simulink model "simulation_environment_thesis"  (R2024b)
settings: Solver=VariableStepAuto  StartTime=0.0  StopTime=3200  RelTol=1e-3
values from neighbouring files (symbols this model uses):
  A = [1, dt; 0, 1]    [estimate_and_control_fully_actuated_with_current.m]
  A1 = [1, 0, 0, 0, dt, 0 ... (first row only)    [estimate_and_control_fully_actuated_with_current.m]
  alpha = 10    [estimate_and_control_fully_actuated_with_current.m]
  alpha_xy_1 = 1    [estimate_and_control_fully_actuated_with_current.m]
  alpha_z_1 = 5    [estimate_and_control_fully_actuated_with_current.m]
  Az1 = [1, dt ... (first row only)    [estimate_and_control_fully_actuated_with_current.m]
  B = [dt; 0]    [estimate_and_control_fully_actuated_with_current.m]
  B1 = [dt, 0 ... (first row only)    [estimate_and_control_fully_actuated_with_current.m]
  Bz1 = [dt ... (first row only)    [estimate_and_control_fully_actuated_with_current.m]
  C1 = [1, 0, 0, 0, 0, 0 ... (first row only)    [estimate_and_control_fully_actuated_with_current.m]
  circumference_x = min_dist_from_ds*cos(theta)    [estimate_and_control_fully_actuated_with_current.m]
  circumference_y = min_dist_from_ds*sin(theta)    [estimate_and_control_fully_actuated_with_current.m]
  const_current = [-r; -s; 0; 0]    [estimate_and_control_fully_actuated_with_current.m]
  csi_psi_1 = 0.0003    [estimate_and_control_fully_actuated_with_current.m]
  csi_r_1 = 0.0003    [estimate_and_control_fully_actuated_with_current.m]
  csi_vcx_1 = 0.0003    [estimate_and_control_fully_actuated_with_current.m]
  csi_vcy_1 = 0.0003    [estimate_and_control_fully_actuated_with_current.m]
  csi_vcz_1 = 0.005    [estimate_and_control_fully_actuated_with_current.m]
  csi_x_1 = 0.003    [estimate_and_control_fully_actuated_with_current.m]
  csi_y_1 = 0.003    [estimate_and_control_fully_actuated_with_current.m]
  csi_z_1 = 0.005    [estimate_and_control_fully_actuated_with_current.m]
  Cz1 = [1, 0]    [estimate_and_control_fully_actuated_with_current.m]
  d = 1    [estimate_and_control_fully_actuated_with_current.m]
  delta_FOV = pi/7    (= 0.448798950513)    [estimate_and_control_fully_actuated_with_current.m]
  desired_speed = speed_0    [estimate_and_control_fully_actuated_with_current.m]
  dt = 1/Tsim    (= 0.005)    [estimate_and_control_fully_actuated_with_current.m]
  eta1 = deg2rad(0.5)    [estimate_and_control_fully_actuated_with_current.m]
  eta2 = deg2rad(0.5)    [estimate_and_control_fully_actuated_with_current.m]
  eta_psi_1 = 0.003    [estimate_and_control_fully_actuated_with_current.m]
  eta_x_1 = 0.03    [estimate_and_control_fully_actuated_with_current.m]
  eta_y_1 = 0.03    [estimate_and_control_fully_actuated_with_current.m]
  eta_z_1 = 0.005    [estimate_and_control_fully_actuated_with_current.m]
  G = dt*eye(2)    [estimate_and_control_fully_actuated_with_current.m]
  G1 = dt*eye(6)    [estimate_and_control_fully_actuated_with_current.m]
  Gz1 = dt*eye(2)    [estimate_and_control_fully_actuated_with_current.m]
  left_reference = L_x*[cos(NED_psi_ds); sin(NED_psi_ds); 0] + L_y_l*[-sin(NED_psi_ds); cos(NED_psi_ds); 0]    [estimate_and_control_fully_actuated_with_current.m]
  max_dist_from_ship = 60    [estimate_and_control_fully_actuated_with_current.m]
  min_dist_from_ds = 45    [estimate_and_control_fully_actuated_with_current.m]
  mu = -5    [estimate_and_control_fully_actuated_with_current.m]
  NED_psi_ds = -pi/2    (= -1.57079632679)    [estimate_and_control_fully_actuated_with_current.m]
  p_b1 = [-90; 90; 5]    [estimate_and_control_fully_actuated_with_current.m]
  position_t_0 = [0; 0; 0; 0]    [estimate_and_control_fully_actuated_with_current.m]
  psi_P = linspace(0,2*pi,360)'    [estimate_and_control_fully_actuated_with_current.m]
  Q = [csi1_kf^2, 0 ... (first row only)    [estimate_and_control_fully_actuated_with_current.m]
  Q1 = [csi_x_1_kf^2, 0, 0, 0, 0, 0 ... (first row only)    [estimate_and_control_fully_actuated_with_current.m]
  Qz1 = [csi_z_1_kf^2, 0 ... (first row only)    [estimate_and_control_fully_actuated_with_current.m]
  R = [eta1_kf^2, 0 ... (first row only)    [estimate_and_control_fully_actuated_with_current.m]
  R1 = [eta_x_1_kf^2, 0, 0 ... (first row only)    [estimate_and_control_fully_actuated_with_current.m]
  right_reference = L_x*[cos(NED_psi_ds); sin(NED_psi_ds); 0] + L_y_r*[sin(NED_psi_ds); -cos(NED_psi_ds); 0]    [estimate_and_control_fully_actuated_with_current.m]
  Rz1 = eta_z_1_kf^2    (= 1e-06)    [estimate_and_control_fully_actuated_with_current.m]
  speed_0 = [0.1, 0.1, 0, 0]'    [estimate_and_control_fully_actuated_with_current.m]
  tau_heave_sat = 30    [estimate_and_control_fully_actuated_with_current.m]
  tau_surge_sat = 10    [estimate_and_control_fully_actuated_with_current.m]
  tau_sway_sat = 30    [estimate_and_control_fully_actuated_with_current.m]
  tau_yaw_sat = 0.1    [estimate_and_control_fully_actuated_with_current.m]
  var_vm_x = 0.001    [estimate_and_control_fully_actuated_with_current.m]
  var_vm_y = 0.001    [estimate_and_control_fully_actuated_with_current.m]
  var_vm_z = 0.001    [estimate_and_control_fully_actuated_with_current.m]
undefined symbols (not defined in the file or in its folder): A2, alpha2, B2, csi1_2, csi2_2, csi3_2, csi4_2, csi5_2, csi6_2, csi7_2, csi8_2, eta1_2, eta2_2, eta3_2, eta4_2, eta5_2, eta6_2, G2, Q2, R2
libraries: simulink x60, simulink_extras x5
(part 7 of 16)

== system simulation_environment_thesis/PHASE 1.21/CONTROLLER1/ANTIWINDUP SURGE ==
  in : Inport
  K : Inport  Port=2
  K1 : Inport  Port=3
  K_t_u : Inport  Port=4
  Integrator21 : Integrator
  MATLAB Function21 : MATLAB Function  TreatAsAtomicUnit=on
  MATLAB Function22 : MATLAB Function  TreatAsAtomicUnit=on
  MATLAB Function23 : MATLAB Function  TreatAsAtomicUnit=on
  Saturation2 : Saturate  UpperLimit=tau_surge_sat  LowerLimit=-tau_surge_sat
  Sum11 : Sum  Inputs=|++
  Sum12 : Sum  Inputs=|+-
  Sum13 : Sum  Inputs=|++
  tau_u : Outport
  wires:
  in/1 -> MATLAB Function22/1(in), MATLAB Function21/1(in)
  Sum12/1 -> MATLAB Function23/1(e_s_u)
  Sum11/1 -> Integrator21/1
  Sum13/1 -> Saturation2/1, Sum12/2
  MATLAB Function22/1(y) -> Sum11/1
  MATLAB Function21/1(y) -> Sum13/1
  Saturation2/1 -> tau_u/1, Sum12/1
  K1/1 -> MATLAB Function21/2(K)
  K_t_u/1 -> MATLAB Function23/2(K_t_u)
  K/1 -> MATLAB Function22/2(K)
  MATLAB Function23/1(y) -> Sum11/2
  Integrator21/1 -> Sum13/2

== system simulation_environment_thesis/PHASE 1.21/CONTROLLER1/ANTIWINDUP SWAY ==
  in : Inport
  K : Inport  Port=2
  K1 : Inport  Port=3
  K_t_v : Inport  Port=4
  Integrator21 : Integrator
  MATLAB Function21 : MATLAB Function  TreatAsAtomicUnit=on
  MATLAB Function22 : MATLAB Function  TreatAsAtomicUnit=on
  MATLAB Function23 : MATLAB Function  TreatAsAtomicUnit=on
  Saturation2 : Saturate  UpperLimit=tau_sway_sat  LowerLimit=-tau_sway_sat
  Sum11 : Sum  Inputs=|++
  Sum12 : Sum  Inputs=|+-
  Sum13 : Sum  Inputs=|++
  tau_v : Outport
  wires:
  in/1 -> MATLAB Function22/1(in), MATLAB Function21/1(in)
  Sum12/1 -> MATLAB Function23/1(e_s_v)
  Sum11/1 -> Integrator21/1
  Sum13/1 -> Saturation2/1, Sum12/2
  MATLAB Function22/1(y) -> Sum11/1
  MATLAB Function21/1(y) -> Sum13/1
  Saturation2/1 -> tau_v/1, Sum12/1
  K1/1 -> MATLAB Function21/2(K)
  K_t_v/1 -> MATLAB Function23/2(K_t_v)
  K/1 -> MATLAB Function22/2(K)
  MATLAB Function23/1(y) -> Sum11/2
  Integrator21/1 -> Sum13/2

== system simulation_environment_thesis/PHASE 1.21/CONTROLLER1/ANTIWINDUP_HEAVE ==
  K1 : Inport
  K2 : Inport  Port=2
  K_t_w : Inport  Port=3
  in1 : Inport  Port=4
  in : Inport  Port=5
  K : Inport  Port=6
  Integrator2 : Integrator
  MATLAB Function11 : MATLAB Function  TreatAsAtomicUnit=on
  MATLAB Function14 : MATLAB Function  TreatAsAtomicUnit=on
  MATLAB Function15 : MATLAB Function  TreatAsAtomicUnit=on
  MATLAB Function16 : MATLAB Function  TreatAsAtomicUnit=on
  Saturation1 : Saturate  UpperLimit=tau_heave_sat  LowerLimit=-tau_heave_sat
  Sum4 : Sum  Inputs=|+-
  Sum8 : Sum  Inputs=|+++
  Sum9 : Sum  Inputs=|++
  tau_w : Outport
  wires:
  in/1 -> MATLAB Function16/1(in)
  MATLAB Function11/1(y) -> Sum9/2
  MATLAB Function14/1(y) -> Sum8/1
  MATLAB Function16/1(y) -> Sum8/3
  K_t_w/1 -> MATLAB Function11/2(K_t_w)
  MATLAB Function15/1(y) -> Sum9/1
  Integrator2/1 -> Sum8/2
  K/1 -> MATLAB Function16/2(K)
  K2/1 -> MATLAB Function14/2(K)
  K1/1 -> MATLAB Function15/2(K)
  Saturation1/1 -> tau_w/1, Sum4/1
  Sum4/1 -> MATLAB Function11/1(e_s_w)
  in1/1 -> MATLAB Function15/1(in), MATLAB Function14/1(in)
  Sum9/1 -> Integrator2/1
  Sum8/1 -> Sum4/2, Saturation1/1

== system simulation_environment_thesis/PHASE 1.21/CONTROLLER1/ANTIWINDUP_YAW ==
  K1 : Inport
  K2 : Inport  Port=2
  K_t_psi : Inport  Port=3
  in1 : Inport  Port=4
  in : Inport  Port=5
  K : Inport  Port=6
  Integrator2 : Integrator
  MATLAB Function11 : MATLAB Function  TreatAsAtomicUnit=on
  MATLAB Function14 : MATLAB Function  TreatAsAtomicUnit=on
  MATLAB Function15 : MATLAB Function  TreatAsAtomicUnit=on
  MATLAB Function16 : MATLAB Function  TreatAsAtomicUnit=on
  Saturation1 : Saturate  UpperLimit=tau_yaw_sat  LowerLimit=-tau_yaw_sat
  Sum4 : Sum  Inputs=|+-
  Sum8 : Sum  Inputs=|+++
  Sum9 : Sum  Inputs=|++
  tau_psi : Outport
  wires:
  MATLAB Function15/1(y) -> Sum9/1
  Sum8/1 -> Sum4/2, Saturation1/1
  in1/1 -> MATLAB Function15/1(in), MATLAB Function14/1(in)
  Saturation1/1 -> tau_psi/1, Sum4/1
  Sum9/1 -> Integrator2/1
  MATLAB Function14/1(y) -> Sum8/1
  MATLAB Function11/1(y) -> Sum9/2
  Integrator2/1 -> Sum8/2
  Sum4/1 -> MATLAB Function11/1(e_s_psi)
  K_t_psi/1 -> MATLAB Function11/2(K_t_psi)
  K1/1 -> MATLAB Function15/2(K)
  K2/1 -> MATLAB Function14/2(K)
  MATLAB Function16/1(y) -> Sum8/3
  in/1 -> MATLAB Function16/1(in)
  K/1 -> MATLAB Function16/2(K)

== system simulation_environment_thesis/PHASE 1.22/CONTROLLER1/ANTIWINDUP SURGE ==
  in : Inport
  K : Inport  Port=2
  K1 : Inport  Port=3
  K_t_u : Inport  Port=4
  Integrator21 : Integrator
  MATLAB Function21 : MATLAB Function  TreatAsAtomicUnit=on
  MATLAB Function22 : MATLAB Function  TreatAsAtomicUnit=on
  MATLAB Function23 : MATLAB Function  TreatAsAtomicUnit=on
  Saturation2 : Saturate  UpperLimit=tau_surge_sat  LowerLimit=-tau_surge_sat
  Sum11 : Sum  Inputs=|++
  Sum12 : Sum  Inputs=|+-
  Sum13 : Sum  Inputs=|++
  tau_u : Outport
  wires:
  in/1 -> MATLAB Function22/1(in), MATLAB Function21/1(in)
  Sum12/1 -> MATLAB Function23/1(e_s_u)
  Sum11/1 -> Integrator21/1
  Sum13/1 -> Saturation2/1, Sum12/2
  MATLAB Function22/1(y) -> Sum11/1
  MATLAB Function21/1(y) -> Sum13/1
  Saturation2/1 -> tau_u/1, Sum12/1
  K1/1 -> MATLAB Function21/2(K)
  K_t_u/1 -> MATLAB Function23/2(K_t_u)
  K/1 -> MATLAB Function22/2(K)
  MATLAB Function23/1(y) -> Sum11/2
  Integrator21/1 -> Sum13/2

== system simulation_environment_thesis/PHASE 1.22/CONTROLLER1/ANTIWINDUP SWAY ==
  in : Inport
  K : Inport  Port=2
  K1 : Inport  Port=3
  K_t_v : Inport  Port=4
  Integrator21 : Integrator
  MATLAB Function21 : MATLAB Function  TreatAsAtomicUnit=on
  MATLAB Function22 : MATLAB Function  TreatAsAtomicUnit=on
  MATLAB Function23 : MATLAB Function  TreatAsAtomicUnit=on
  Saturation2 : Saturate  UpperLimit=tau_sway_sat  LowerLimit=-tau_sway_sat
  Sum11 : Sum  Inputs=|++
  Sum12 : Sum  Inputs=|+-
  Sum13 : Sum  Inputs=|++
  tau_v : Outport
  wires:
  in/1 -> MATLAB Function22/1(in), MATLAB Function21/1(in)
  Sum12/1 -> MATLAB Function23/1(e_s_v)
  Sum11/1 -> Integrator21/1
  Sum13/1 -> Saturation2/1, Sum12/2
  MATLAB Function22/1(y) -> Sum11/1
  MATLAB Function21/1(y) -> Sum13/1
  Saturation2/1 -> tau_v/1, Sum12/1
  K1/1 -> MATLAB Function21/2(K)
  K_t_v/1 -> MATLAB Function23/2(K_t_v)
  K/1 -> MATLAB Function22/2(K)
  MATLAB Function23/1(y) -> Sum11/2
  Integrator21/1 -> Sum13/2

== system simulation_environment_thesis/PHASE 1.22/CONTROLLER1/ANTIWINDUP_HEAVE ==
  K1 : Inport
  K2 : Inport  Port=2
  K_t_w : Inport  Port=3
  in1 : Inport  Port=4
  in : Inport  Port=5
  K : Inport  Port=6
  Integrator2 : Integrator
  MATLAB Function11 : MATLAB Function  TreatAsAtomicUnit=on
  MATLAB Function14 : MATLAB Function  TreatAsAtomicUnit=on
  MATLAB Function15 : MATLAB Function  TreatAsAtomicUnit=on
  MATLAB Function16 : MATLAB Function  TreatAsAtomicUnit=on
  Saturation1 : Saturate  UpperLimit=tau_heave_sat  LowerLimit=-tau_heave_sat
  Sum4 : Sum  Inputs=|+-
  Sum8 : Sum  Inputs=|+++
  Sum9 : Sum  Inputs=|++
  tau_w : Outport
  wires:
  in/1 -> MATLAB Function16/1(in)
  MATLAB Function11/1(y) -> Sum9/2
  MATLAB Function14/1(y) -> Sum8/1
  MATLAB Function16/1(y) -> Sum8/3
  K_t_w/1 -> MATLAB Function11/2(K_t_w)
  MATLAB Function15/1(y) -> Sum9/1
  Integrator2/1 -> Sum8/2
  K/1 -> MATLAB Function16/2(K)
  K2/1 -> MATLAB Function14/2(K)
  K1/1 -> MATLAB Function15/2(K)
  Saturation1/1 -> tau_w/1, Sum4/1
  Sum4/1 -> MATLAB Function11/1(e_s_w)
  in1/1 -> MATLAB Function15/1(in), MATLAB Function14/1(in)
  Sum9/1 -> Integrator2/1
  Sum8/1 -> Sum4/2, Saturation1/1

== system simulation_environment_thesis/PHASE 1.22/CONTROLLER1/ANTIWINDUP_YAW ==
  K1 : Inport
  K2 : Inport  Port=2
  K_t_psi : Inport  Port=3
  in1 : Inport  Port=4
  in : Inport  Port=5
  K : Inport  Port=6
  Integrator2 : Integrator
  MATLAB Function11 : MATLAB Function  TreatAsAtomicUnit=on
  MATLAB Function14 : MATLAB Function  TreatAsAtomicUnit=on
  MATLAB Function15 : MATLAB Function  TreatAsAtomicUnit=on
  MATLAB Function16 : MATLAB Function  TreatAsAtomicUnit=on
  Saturation1 : Saturate  UpperLimit=tau_yaw_sat  LowerLimit=-tau_yaw_sat
  Sum4 : Sum  Inputs=|+-
  Sum8 : Sum  Inputs=|+++
  Sum9 : Sum  Inputs=|++
  tau_psi : Outport
  wires:
  MATLAB Function15/1(y) -> Sum9/1
  Sum8/1 -> Sum4/2, Saturation1/1
  in1/1 -> MATLAB Function15/1(in), MATLAB Function14/1(in)
  Saturation1/1 -> tau_psi/1, Sum4/1
  Sum9/1 -> Integrator2/1
  MATLAB Function14/1(y) -> Sum8/1
  MATLAB Function11/1(y) -> Sum9/2
  Integrator2/1 -> Sum8/2
  Sum4/1 -> MATLAB Function11/1(e_s_psi)
  K_t_psi/1 -> MATLAB Function11/2(K_t_psi)
  K1/1 -> MATLAB Function15/2(K)
  K2/1 -> MATLAB Function14/2(K)
  MATLAB Function16/1(y) -> Sum8/3
  in/1 -> MATLAB Function16/1(in)
  K/1 -> MATLAB Function16/2(K)

== system simulation_environment_thesis/PHASE 1.24/CONTROLLER1/ANTIWINDUP SURGE ==
  in : Inport
  K : Inport  Port=2
  K1 : Inport  Port=3
  K_t_u : Inport  Port=4
  Integrator21 : Integrator
  MATLAB Function21 : MATLAB Function  TreatAsAtomicUnit=on
  MATLAB Function22 : MATLAB Function  TreatAsAtomicUnit=on
  MATLAB Function23 : MATLAB Function  TreatAsAtomicUnit=on
  Saturation2 : Saturate  UpperLimit=tau_surge_sat  LowerLimit=-tau_surge_sat
  Sum11 : Sum  Inputs=|++
  Sum12 : Sum  Inputs=|+-
  Sum13 : Sum  Inputs=|++
  tau_u : Outport
  wires:
  in/1 -> MATLAB Function22/1(in), MATLAB Function21/1(in)
  Sum12/1 -> MATLAB Function23/1(e_s_u)
  Sum11/1 -> Integrator21/1
  Sum13/1 -> Saturation2/1, Sum12/2
  MATLAB Function22/1(y) -> Sum11/1
  MATLAB Function21/1(y) -> Sum13/1
  Saturation2/1 -> tau_u/1, Sum12/1
  K1/1 -> MATLAB Function21/2(K)
  K_t_u/1 -> MATLAB Function23/2(K_t_u)
  K/1 -> MATLAB Function22/2(K)
  MATLAB Function23/1(y) -> Sum11/2
  Integrator21/1 -> Sum13/2

== system simulation_environment_thesis/PHASE 1.24/CONTROLLER1/ANTIWINDUP SWAY ==
  in : Inport
  K : Inport  Port=2
  K1 : Inport  Port=3
  K_t_v : Inport  Port=4
  Integrator21 : Integrator
  MATLAB Function21 : MATLAB Function  TreatAsAtomicUnit=on
  MATLAB Function22 : MATLAB Function  TreatAsAtomicUnit=on
  MATLAB Function23 : MATLAB Function  TreatAsAtomicUnit=on
  Saturation2 : Saturate  UpperLimit=tau_sway_sat  LowerLimit=-tau_sway_sat
  Sum11 : Sum  Inputs=|++
  Sum12 : Sum  Inputs=|+-
  Sum13 : Sum  Inputs=|++
  tau_v : Outport
  wires:
  in/1 -> MATLAB Function22/1(in), MATLAB Function21/1(in)
  Sum12/1 -> MATLAB Function23/1(e_s_v)
  Sum11/1 -> Integrator21/1
  Sum13/1 -> Saturation2/1, Sum12/2
  MATLAB Function22/1(y) -> Sum11/1
  MATLAB Function21/1(y) -> Sum13/1
  Saturation2/1 -> tau_v/1, Sum12/1
  K1/1 -> MATLAB Function21/2(K)
  K_t_v/1 -> MATLAB Function23/2(K_t_v)
  K/1 -> MATLAB Function22/2(K)
  MATLAB Function23/1(y) -> Sum11/2
  Integrator21/1 -> Sum13/2

== system simulation_environment_thesis/PHASE 1.24/CONTROLLER1/ANTIWINDUP_HEAVE ==
  K1 : Inport
  K2 : Inport  Port=2
  K_t_w : Inport  Port=3
  in1 : Inport  Port=4
  in : Inport  Port=5
  K : Inport  Port=6
  Integrator2 : Integrator
  MATLAB Function11 : MATLAB Function  TreatAsAtomicUnit=on
  MATLAB Function14 : MATLAB Function  TreatAsAtomicUnit=on
  MATLAB Function15 : MATLAB Function  TreatAsAtomicUnit=on
  MATLAB Function16 : MATLAB Function  TreatAsAtomicUnit=on
  Saturation1 : Saturate  UpperLimit=tau_heave_sat  LowerLimit=-tau_heave_sat
  Sum4 : Sum  Inputs=|+-
  Sum8 : Sum  Inputs=|+++
  Sum9 : Sum  Inputs=|++
  tau_w : Outport
  wires:
  in/1 -> MATLAB Function16/1(in)
  MATLAB Function11/1(y) -> Sum9/2
  MATLAB Function14/1(y) -> Sum8/1
  MATLAB Function16/1(y) -> Sum8/3
  K_t_w/1 -> MATLAB Function11/2(K_t_w)
  MATLAB Function15/1(y) -> Sum9/1
  Integrator2/1 -> Sum8/2
  K/1 -> MATLAB Function16/2(K)
  K2/1 -> MATLAB Function14/2(K)
  K1/1 -> MATLAB Function15/2(K)
  Saturation1/1 -> tau_w/1, Sum4/1
  Sum4/1 -> MATLAB Function11/1(e_s_w)
  in1/1 -> MATLAB Function15/1(in), MATLAB Function14/1(in)
  Sum9/1 -> Integrator2/1
  Sum8/1 -> Sum4/2, Saturation1/1

== system simulation_environment_thesis/PHASE 1.24/CONTROLLER1/ANTIWINDUP_YAW ==
  K1 : Inport
  K2 : Inport  Port=2
  K_t_psi : Inport  Port=3
  in1 : Inport  Port=4
  in : Inport  Port=5
  K : Inport  Port=6
  Integrator2 : Integrator
  MATLAB Function11 : MATLAB Function  TreatAsAtomicUnit=on
  MATLAB Function14 : MATLAB Function  TreatAsAtomicUnit=on
  MATLAB Function15 : MATLAB Function  TreatAsAtomicUnit=on
  MATLAB Function16 : MATLAB Function  TreatAsAtomicUnit=on
  Saturation1 : Saturate  UpperLimit=tau_yaw_sat  LowerLimit=-tau_yaw_sat
  Sum4 : Sum  Inputs=|+-
  Sum8 : Sum  Inputs=|+++
  Sum9 : Sum  Inputs=|++
  tau_psi : Outport
  wires:
  MATLAB Function15/1(y) -> Sum9/1
  Sum8/1 -> Sum4/2, Saturation1/1
  in1/1 -> MATLAB Function15/1(in), MATLAB Function14/1(in)
  Saturation1/1 -> tau_psi/1, Sum4/1
  Sum9/1 -> Integrator2/1
  MATLAB Function14/1(y) -> Sum8/1
  MATLAB Function11/1(y) -> Sum9/2
  Integrator2/1 -> Sum8/2
  Sum4/1 -> MATLAB Function11/1(e_s_psi)
  K_t_psi/1 -> MATLAB Function11/2(K_t_psi)
  K1/1 -> MATLAB Function15/2(K)
  K2/1 -> MATLAB Function14/2(K)
  MATLAB Function16/1(y) -> Sum8/3
  in/1 -> MATLAB Function16/1(in)
  K/1 -> MATLAB Function16/2(K)

== system simulation_environment_thesis/PHASE 2  /CONTROLLER/ANTIWINDUP SURGE ==
  in : Inport
  K : Inport  Port=2
  K1 : Inport  Port=3
  K_t_u : Inport  Port=4
  Integrator21 : Integrator
  MATLAB Function21 : MATLAB Function  TreatAsAtomicUnit=on
  MATLAB Function22 : MATLAB Function  TreatAsAtomicUnit=on
  MATLAB Function23 : MATLAB Function  TreatAsAtomicUnit=on
  Saturation2 : Saturate  UpperLimit=tau_surge_sat  LowerLimit=-tau_surge_sat
  Sum11 : Sum  Inputs=|++
  Sum12 : Sum  Inputs=|+-
  Sum13 : Sum  Inputs=|++
  tau_u : Outport
  wires:
  Sum11/1 -> Integrator21/1
  in/1 -> MATLAB Function21/1(in), MATLAB Function22/1(in)
  Sum12/1 -> MATLAB Function23/1(e_s_u)
  Integrator21/1 -> Sum13/2
  Sum13/1 -> Saturation2/1, Sum12/2
  MATLAB Function22/1(y) -> Sum11/1
  MATLAB Function23/1(y) -> Sum11/2
  MATLAB Function21/1(y) -> Sum13/1
  Saturation2/1 -> tau_u/1, Sum12/1
  K1/1 -> MATLAB Function21/2(K)
  K_t_u/1 -> MATLAB Function23/2(K_t_u)
  K/1 -> MATLAB Function22/2(K)

== system simulation_environment_thesis/PHASE 2  /CONTROLLER/ANTIWINDUP SWAY ==
  in : Inport
  K : Inport  Port=2
  K1 : Inport  Port=3
  K_t_v : Inport  Port=4
  Integrator21 : Integrator
  MATLAB Function21 : MATLAB Function  TreatAsAtomicUnit=on
  MATLAB Function22 : MATLAB Function  TreatAsAtomicUnit=on
  MATLAB Function23 : MATLAB Function  TreatAsAtomicUnit=on
  Saturation2 : Saturate  UpperLimit=tau_sway_sat  LowerLimit=-tau_sway_sat
  Sum11 : Sum  Inputs=|++
  Sum12 : Sum  Inputs=|+-
  Sum13 : Sum  Inputs=|++
  tau_v : Outport
  wires:
  Sum11/1 -> Integrator21/1
  in/1 -> MATLAB Function22/1(in), MATLAB Function21/1(in)
  Sum12/1 -> MATLAB Function23/1(e_s_v)
  Integrator21/1 -> Sum13/2
  Sum13/1 -> Saturation2/1, Sum12/2
  MATLAB Function22/1(y) -> Sum11/1
  MATLAB Function23/1(y) -> Sum11/2
  MATLAB Function21/1(y) -> Sum13/1
  Saturation2/1 -> tau_v/1, Sum12/1
  K1/1 -> MATLAB Function21/2(K)
  K_t_v/1 -> MATLAB Function23/2(K_t_v)
  K/1 -> MATLAB Function22/2(K)

== system simulation_environment_thesis/PHASE 2  /CONTROLLER/ANTIWINDUP_HEAVE ==
  K1 : Inport
  K2 : Inport  Port=2
  K_t_w : Inport  Port=3
  in1 : Inport  Port=4
  in : Inport  Port=5
  K : Inport  Port=6
  Integrator2 : Integrator
  MATLAB Function11 : MATLAB Function  TreatAsAtomicUnit=on
  MATLAB Function14 : MATLAB Function  TreatAsAtomicUnit=on
  MATLAB Function15 : MATLAB Function  TreatAsAtomicUnit=on
  MATLAB Function16 : MATLAB Function  TreatAsAtomicUnit=on
  Saturation1 : Saturate  UpperLimit=tau_heave_sat  LowerLimit=-tau_heave_sat
  Sum4 : Sum  Inputs=|+-
  Sum8 : Sum  Inputs=|+++
  Sum9 : Sum  Inputs=|++
  tau_w : Outport
  wires:
  in/1 -> MATLAB Function16/1(in)
  MATLAB Function11/1(y) -> Sum9/2
  MATLAB Function14/1(y) -> Sum8/1
  MATLAB Function16/1(y) -> Sum8/3
  K_t_w/1 -> MATLAB Function11/2(K_t_w)
  MATLAB Function15/1(y) -> Sum9/1
  Integrator2/1 -> Sum8/2
  K/1 -> MATLAB Function16/2(K)
  K2/1 -> MATLAB Function14/2(K)
  K1/1 -> MATLAB Function15/2(K)
  Saturation1/1 -> tau_w/1, Sum4/1
  Sum4/1 -> MATLAB Function11/1(e_s_w)
  in1/1 -> MATLAB Function15/1(in), MATLAB Function14/1(in)
  Sum9/1 -> Integrator2/1
  Sum8/1 -> Sum4/2, Saturation1/1

== system simulation_environment_thesis/PHASE 2  /CONTROLLER/ANTIWINDUP_YAW ==
  K1 : Inport
  K2 : Inport  Port=2
  K_t_psi : Inport  Port=3
  in1 : Inport  Port=4
  in : Inport  Port=5
  K : Inport  Port=6
  Integrator2 : Integrator
  MATLAB Function11 : MATLAB Function  TreatAsAtomicUnit=on
  MATLAB Function14 : MATLAB Function  TreatAsAtomicUnit=on
  MATLAB Function15 : MATLAB Function  TreatAsAtomicUnit=on
  MATLAB Function16 : MATLAB Function  TreatAsAtomicUnit=on
  Saturation1 : Saturate  UpperLimit=tau_yaw_sat  LowerLimit=-tau_yaw_sat
  Sum4 : Sum  Inputs=|+-
  Sum8 : Sum  Inputs=|+++
  Sum9 : Sum  Inputs=|++
  tau_psi : Outport
  wires:
  MATLAB Function15/1(y) -> Sum9/1
  Sum8/1 -> Sum4/2, Saturation1/1
  in1/1 -> MATLAB Function15/1(in), MATLAB Function14/1(in)
  Saturation1/1 -> tau_psi/1, Sum4/1
  Sum9/1 -> Integrator2/1
  MATLAB Function14/1(y) -> Sum8/1
  MATLAB Function11/1(y) -> Sum9/2
  Integrator2/1 -> Sum8/2
  Sum4/1 -> MATLAB Function11/1(e_s_psi)
  K_t_psi/1 -> MATLAB Function11/2(K_t_psi)
  K1/1 -> MATLAB Function15/2(K)
  K2/1 -> MATLAB Function14/2(K)
  MATLAB Function16/1(y) -> Sum8/3
  in/1 -> MATLAB Function16/1(in)
  K/1 -> MATLAB Function16/2(K)

== system simulation_environment_thesis/PHASE 2.21/CONTROLLER4/ANTIWINDUP SURGE ==
  in : Inport
  K : Inport  Port=2
  K1 : Inport  Port=3
  K_t_u : Inport  Port=4
  Integrator21 : Integrator
  MATLAB Function21 : MATLAB Function  TreatAsAtomicUnit=on
  MATLAB Function22 : MATLAB Function  TreatAsAtomicUnit=on
  MATLAB Function23 : MATLAB Function  TreatAsAtomicUnit=on
  Saturation2 : Saturate  UpperLimit=tau_surge_sat  LowerLimit=-tau_surge_sat
  Sum11 : Sum  Inputs=|++
  Sum12 : Sum  Inputs=|+-
  Sum13 : Sum  Inputs=|++
  tau_u : Outport
  wires:
  Sum11/1 -> Integrator21/1
  in/1 -> MATLAB Function21/1(in), MATLAB Function22/1(in)
  Sum12/1 -> MATLAB Function23/1(e_s_u)
  Integrator21/1 -> Sum13/2
  Sum13/1 -> Saturation2/1, Sum12/2
  MATLAB Function22/1(y) -> Sum11/1
  MATLAB Function23/1(y) -> Sum11/2
  MATLAB Function21/1(y) -> Sum13/1
  Saturation2/1 -> tau_u/1, Sum12/1
  K1/1 -> MATLAB Function21/2(K)
  K_t_u/1 -> MATLAB Function23/2(K_t_u)
  K/1 -> MATLAB Function22/2(K)

== system simulation_environment_thesis/PHASE 2.21/CONTROLLER4/ANTIWINDUP SWAY ==
  in : Inport
  K : Inport  Port=2
  K1 : Inport  Port=3
  K_t_v : Inport  Port=4
  Integrator21 : Integrator
  MATLAB Function21 : MATLAB Function  TreatAsAtomicUnit=on
  MATLAB Function22 : MATLAB Function  TreatAsAtomicUnit=on
  MATLAB Function23 : MATLAB Function  TreatAsAtomicUnit=on
  Saturation2 : Saturate  UpperLimit=tau_sway_sat  LowerLimit=-tau_sway_sat
  Sum11 : Sum  Inputs=|++
  Sum12 : Sum  Inputs=|+-
  Sum13 : Sum  Inputs=|++
  tau_v : Outport
  wires:
  Sum11/1 -> Integrator21/1
  in/1 -> MATLAB Function22/1(in), MATLAB Function21/1(in)
  Sum12/1 -> MATLAB Function23/1(e_s_v)
  Integrator21/1 -> Sum13/2
  Sum13/1 -> Saturation2/1, Sum12/2
  MATLAB Function22/1(y) -> Sum11/1
  MATLAB Function23/1(y) -> Sum11/2
  MATLAB Function21/1(y) -> Sum13/1
  Saturation2/1 -> tau_v/1, Sum12/1
  K1/1 -> MATLAB Function21/2(K)
  K_t_v/1 -> MATLAB Function23/2(K_t_v)
  K/1 -> MATLAB Function22/2(K)

== system simulation_environment_thesis/PHASE 2.21/CONTROLLER4/ANTIWINDUP_HEAVE ==
  K1 : Inport
  K2 : Inport  Port=2
  K_t_w : Inport  Port=3
  in1 : Inport  Port=4
  in : Inport  Port=5
  K : Inport  Port=6
  Integrator2 : Integrator
  MATLAB Function11 : MATLAB Function  TreatAsAtomicUnit=on
  MATLAB Function14 : MATLAB Function  TreatAsAtomicUnit=on
  MATLAB Function15 : MATLAB Function  TreatAsAtomicUnit=on
  MATLAB Function16 : MATLAB Function  TreatAsAtomicUnit=on
  Saturation1 : Saturate  UpperLimit=tau_heave_sat  LowerLimit=-tau_heave_sat
  Sum4 : Sum  Inputs=|+-
  Sum8 : Sum  Inputs=|+++
  Sum9 : Sum  Inputs=|++
  tau_w : Outport
  wires:
  in/1 -> MATLAB Function16/1(in)
  MATLAB Function11/1(y) -> Sum9/2
  MATLAB Function14/1(y) -> Sum8/1
  MATLAB Function16/1(y) -> Sum8/3
  K_t_w/1 -> MATLAB Function11/2(K_t_w)
  MATLAB Function15/1(y) -> Sum9/1
  Integrator2/1 -> Sum8/2
  K/1 -> MATLAB Function16/2(K)
  K2/1 -> MATLAB Function14/2(K)
  K1/1 -> MATLAB Function15/2(K)
  Saturation1/1 -> tau_w/1, Sum4/1
  Sum4/1 -> MATLAB Function11/1(e_s_w)
  in1/1 -> MATLAB Function15/1(in), MATLAB Function14/1(in)
  Sum9/1 -> Integrator2/1
  Sum8/1 -> Sum4/2, Saturation1/1

== system simulation_environment_thesis/PHASE 2.21/CONTROLLER4/ANTIWINDUP_YAW ==
  K1 : Inport
  K2 : Inport  Port=2
  K_t_psi : Inport  Port=3
  in1 : Inport  Port=4
  in : Inport  Port=5
  K : Inport  Port=6
  Integrator2 : Integrator
  MATLAB Function11 : MATLAB Function  TreatAsAtomicUnit=on
  MATLAB Function14 : MATLAB Function  TreatAsAtomicUnit=on
  MATLAB Function15 : MATLAB Function  TreatAsAtomicUnit=on
  MATLAB Function16 : MATLAB Function  TreatAsAtomicUnit=on
  Saturation1 : Saturate  UpperLimit=tau_yaw_sat  LowerLimit=-tau_yaw_sat
  Sum4 : Sum  Inputs=|+-
  Sum8 : Sum  Inputs=|+++
  Sum9 : Sum  Inputs=|++
  tau_psi : Outport
  wires:
  MATLAB Function15/1(y) -> Sum9/1
  Sum8/1 -> Sum4/2, Saturation1/1
  in1/1 -> MATLAB Function15/1(in), MATLAB Function14/1(in)
  Saturation1/1 -> tau_psi/1, Sum4/1
  Sum9/1 -> Integrator2/1
  MATLAB Function14/1(y) -> Sum8/1
  MATLAB Function11/1(y) -> Sum9/2
  Integrator2/1 -> Sum8/2
  Sum4/1 -> MATLAB Function11/1(e_s_psi)
  K_t_psi/1 -> MATLAB Function11/2(K_t_psi)
  K1/1 -> MATLAB Function15/2(K)
  K2/1 -> MATLAB Function14/2(K)
  MATLAB Function16/1(y) -> Sum8/3
  in/1 -> MATLAB Function16/1(in)
  K/1 -> MATLAB Function16/2(K)

== system simulation_environment_thesis/PHASE 2.22/CONTROLLER4/ANTIWINDUP SURGE ==
  in : Inport
  K : Inport  Port=2
  K1 : Inport  Port=3
  K_t_u : Inport  Port=4
  Integrator21 : Integrator
  MATLAB Function21 : MATLAB Function  TreatAsAtomicUnit=on
  MATLAB Function22 : MATLAB Function  TreatAsAtomicUnit=on
  MATLAB Function23 : MATLAB Function  TreatAsAtomicUnit=on
  Saturation2 : Saturate  UpperLimit=tau_surge_sat  LowerLimit=-tau_surge_sat
  Sum11 : Sum  Inputs=|++
  Sum12 : Sum  Inputs=|+-
  Sum13 : Sum  Inputs=|++
  tau_u : Outport
  wires:
  Sum11/1 -> Integrator21/1
  in/1 -> MATLAB Function21/1(in), MATLAB Function22/1(in)
  Sum12/1 -> MATLAB Function23/1(e_s_u)
  Integrator21/1 -> Sum13/2
  Sum13/1 -> Saturation2/1, Sum12/2
  MATLAB Function22/1(y) -> Sum11/1
  MATLAB Function23/1(y) -> Sum11/2
  MATLAB Function21/1(y) -> Sum13/1
  Saturation2/1 -> tau_u/1, Sum12/1
  K1/1 -> MATLAB Function21/2(K)
  K_t_u/1 -> MATLAB Function23/2(K_t_u)
  K/1 -> MATLAB Function22/2(K)

== system simulation_environment_thesis/PHASE 2.22/CONTROLLER4/ANTIWINDUP SWAY ==
  in : Inport
  K : Inport  Port=2
  K1 : Inport  Port=3
  K_t_v : Inport  Port=4
  Integrator21 : Integrator
  MATLAB Function21 : MATLAB Function  TreatAsAtomicUnit=on
  MATLAB Function22 : MATLAB Function  TreatAsAtomicUnit=on
  MATLAB Function23 : MATLAB Function  TreatAsAtomicUnit=on
  Saturation2 : Saturate  UpperLimit=tau_sway_sat  LowerLimit=-tau_sway_sat
  Sum11 : Sum  Inputs=|++
  Sum12 : Sum  Inputs=|+-
  Sum13 : Sum  Inputs=|++
  tau_v : Outport
  wires:
  Sum11/1 -> Integrator21/1
  in/1 -> MATLAB Function22/1(in), MATLAB Function21/1(in)
  Sum12/1 -> MATLAB Function23/1(e_s_v)
  Integrator21/1 -> Sum13/2
  Sum13/1 -> Saturation2/1, Sum12/2
  MATLAB Function22/1(y) -> Sum11/1
  MATLAB Function23/1(y) -> Sum11/2
  MATLAB Function21/1(y) -> Sum13/1
  Saturation2/1 -> tau_v/1, Sum12/1
  K1/1 -> MATLAB Function21/2(K)
  K_t_v/1 -> MATLAB Function23/2(K_t_v)
  K/1 -> MATLAB Function22/2(K)

== system simulation_environment_thesis/PHASE 2.22/CONTROLLER4/ANTIWINDUP_HEAVE ==
  K1 : Inport
  K2 : Inport  Port=2
  K_t_w : Inport  Port=3
  in1 : Inport  Port=4
  in : Inport  Port=5
  K : Inport  Port=6
  Integrator2 : Integrator
  MATLAB Function11 : MATLAB Function  TreatAsAtomicUnit=on
  MATLAB Function14 : MATLAB Function  TreatAsAtomicUnit=on
  MATLAB Function15 : MATLAB Function  TreatAsAtomicUnit=on
  MATLAB Function16 : MATLAB Function  TreatAsAtomicUnit=on
  Saturation1 : Saturate  UpperLimit=tau_heave_sat  LowerLimit=-tau_heave_sat
  Sum4 : Sum  Inputs=|+-
  Sum8 : Sum  Inputs=|+++
  Sum9 : Sum  Inputs=|++
  tau_w : Outport
  wires:
  in/1 -> MATLAB Function16/1(in)
  MATLAB Function11/1(y) -> Sum9/2
  MATLAB Function14/1(y) -> Sum8/1
  MATLAB Function16/1(y) -> Sum8/3
  K_t_w/1 -> MATLAB Function11/2(K_t_w)
  MATLAB Function15/1(y) -> Sum9/1
  Integrator2/1 -> Sum8/2
  K/1 -> MATLAB Function16/2(K)
  K2/1 -> MATLAB Function14/2(K)
  K1/1 -> MATLAB Function15/2(K)
  Saturation1/1 -> tau_w/1, Sum4/1
  Sum4/1 -> MATLAB Function11/1(e_s_w)
  in1/1 -> MATLAB Function15/1(in), MATLAB Function14/1(in)
  Sum9/1 -> Integrator2/1
  Sum8/1 -> Sum4/2, Saturation1/1

== system simulation_environment_thesis/PHASE 2.22/CONTROLLER4/ANTIWINDUP_YAW ==
  K1 : Inport
  K2 : Inport  Port=2
  K_t_psi : Inport  Port=3
  in1 : Inport  Port=4
  in : Inport  Port=5
  K : Inport  Port=6
  Integrator2 : Integrator
  MATLAB Function11 : MATLAB Function  TreatAsAtomicUnit=on
  MATLAB Function14 : MATLAB Function  TreatAsAtomicUnit=on
  MATLAB Function15 : MATLAB Function  TreatAsAtomicUnit=on
  MATLAB Function16 : MATLAB Function  TreatAsAtomicUnit=on
  Saturation1 : Saturate  UpperLimit=tau_yaw_sat  LowerLimit=-tau_yaw_sat
  Sum4 : Sum  Inputs=|+-
  Sum8 : Sum  Inputs=|+++
  Sum9 : Sum  Inputs=|++
  tau_psi : Outport
  wires:
  MATLAB Function15/1(y) -> Sum9/1
  Sum8/1 -> Sum4/2, Saturation1/1
  in1/1 -> MATLAB Function15/1(in), MATLAB Function14/1(in)
  Saturation1/1 -> tau_psi/1, Sum4/1
  Sum9/1 -> Integrator2/1
  MATLAB Function14/1(y) -> Sum8/1
  MATLAB Function11/1(y) -> Sum9/2
  Integrator2/1 -> Sum8/2
  Sum4/1 -> MATLAB Function11/1(e_s_psi)
  K_t_psi/1 -> MATLAB Function11/2(K_t_psi)
  K1/1 -> MATLAB Function15/2(K)
  K2/1 -> MATLAB Function14/2(K)
  MATLAB Function16/1(y) -> Sum8/3
  in/1 -> MATLAB Function16/1(in)
  K/1 -> MATLAB Function16/2(K)

== system simulation_environment_thesis/PHASE 2.24/CONTROLLER4/ANTIWINDUP SURGE ==
  in : Inport
  K : Inport  Port=2
  K1 : Inport  Port=3
  K_t_u : Inport  Port=4
  Integrator21 : Integrator
  MATLAB Function21 : MATLAB Function  TreatAsAtomicUnit=on
  MATLAB Function22 : MATLAB Function  TreatAsAtomicUnit=on
  MATLAB Function23 : MATLAB Function  TreatAsAtomicUnit=on
  Saturation2 : Saturate  UpperLimit=tau_surge_sat  LowerLimit=-tau_surge_sat
  Sum11 : Sum  Inputs=|++
  Sum12 : Sum  Inputs=|+-
  Sum13 : Sum  Inputs=|++
  tau_u : Outport
  wires:
  Sum11/1 -> Integrator21/1
  in/1 -> MATLAB Function21/1(in), MATLAB Function22/1(in)
  Sum12/1 -> MATLAB Function23/1(e_s_u)
  Integrator21/1 -> Sum13/2
  Sum13/1 -> Saturation2/1, Sum12/2
  MATLAB Function22/1(y) -> Sum11/1
  MATLAB Function23/1(y) -> Sum11/2
  MATLAB Function21/1(y) -> Sum13/1
  Saturation2/1 -> tau_u/1, Sum12/1
  K1/1 -> MATLAB Function21/2(K)
  K_t_u/1 -> MATLAB Function23/2(K_t_u)
  K/1 -> MATLAB Function22/2(K)

== system simulation_environment_thesis/PHASE 2.24/CONTROLLER4/ANTIWINDUP SWAY ==
  in : Inport
  K : Inport  Port=2
  K1 : Inport  Port=3
  K_t_v : Inport  Port=4
  Integrator21 : Integrator
  MATLAB Function21 : MATLAB Function  TreatAsAtomicUnit=on
  MATLAB Function22 : MATLAB Function  TreatAsAtomicUnit=on
  MATLAB Function23 : MATLAB Function  TreatAsAtomicUnit=on
  Saturation2 : Saturate  UpperLimit=tau_sway_sat  LowerLimit=-tau_sway_sat
  Sum11 : Sum  Inputs=|++
  Sum12 : Sum  Inputs=|+-
  Sum13 : Sum  Inputs=|++
  tau_v : Outport
  wires:
  Sum11/1 -> Integrator21/1
  in/1 -> MATLAB Function22/1(in), MATLAB Function21/1(in)
  Sum12/1 -> MATLAB Function23/1(e_s_v)
  Integrator21/1 -> Sum13/2
  Sum13/1 -> Saturation2/1, Sum12/2
  MATLAB Function22/1(y) -> Sum11/1
  MATLAB Function23/1(y) -> Sum11/2
  MATLAB Function21/1(y) -> Sum13/1
  Saturation2/1 -> tau_v/1, Sum12/1
  K1/1 -> MATLAB Function21/2(K)
  K_t_v/1 -> MATLAB Function23/2(K_t_v)
  K/1 -> MATLAB Function22/2(K)

== system simulation_environment_thesis/PHASE 2.24/CONTROLLER4/ANTIWINDUP_HEAVE ==
  K1 : Inport
  K2 : Inport  Port=2
  K_t_w : Inport  Port=3
  in1 : Inport  Port=4
  in : Inport  Port=5
  K : Inport  Port=6
  Integrator2 : Integrator
  MATLAB Function11 : MATLAB Function  TreatAsAtomicUnit=on
  MATLAB Function14 : MATLAB Function  TreatAsAtomicUnit=on
  MATLAB Function15 : MATLAB Function  TreatAsAtomicUnit=on
  MATLAB Function16 : MATLAB Function  TreatAsAtomicUnit=on
  Saturation1 : Saturate  UpperLimit=tau_heave_sat  LowerLimit=-tau_heave_sat
  Sum4 : Sum  Inputs=|+-
  Sum8 : Sum  Inputs=|+++
  Sum9 : Sum  Inputs=|++
  tau_w : Outport
  wires:
  in/1 -> MATLAB Function16/1(in)
  MATLAB Function11/1(y) -> Sum9/2
  MATLAB Function14/1(y) -> Sum8/1
  MATLAB Function16/1(y) -> Sum8/3
  K_t_w/1 -> MATLAB Function11/2(K_t_w)
  MATLAB Function15/1(y) -> Sum9/1
  Integrator2/1 -> Sum8/2
  K/1 -> MATLAB Function16/2(K)
  K2/1 -> MATLAB Function14/2(K)
  K1/1 -> MATLAB Function15/2(K)
  Saturation1/1 -> tau_w/1, Sum4/1
  Sum4/1 -> MATLAB Function11/1(e_s_w)
  in1/1 -> MATLAB Function15/1(in), MATLAB Function14/1(in)
  Sum9/1 -> Integrator2/1
  Sum8/1 -> Sum4/2, Saturation1/1

== system simulation_environment_thesis/PHASE 2.24/CONTROLLER4/ANTIWINDUP_YAW ==
  K1 : Inport
  K2 : Inport  Port=2
  K_t_psi : Inport  Port=3
  in1 : Inport  Port=4
  in : Inport  Port=5
  K : Inport  Port=6
  Integrator2 : Integrator
  MATLAB Function11 : MATLAB Function  TreatAsAtomicUnit=on
  MATLAB Function14 : MATLAB Function  TreatAsAtomicUnit=on
  MATLAB Function15 : MATLAB Function  TreatAsAtomicUnit=on
  MATLAB Function16 : MATLAB Function  TreatAsAtomicUnit=on
  Saturation1 : Saturate  UpperLimit=tau_yaw_sat  LowerLimit=-tau_yaw_sat
  Sum4 : Sum  Inputs=|+-
  Sum8 : Sum  Inputs=|+++
  Sum9 : Sum  Inputs=|++
  tau_psi : Outport
  wires:
  MATLAB Function15/1(y) -> Sum9/1
  Sum8/1 -> Sum4/2, Saturation1/1
  in1/1 -> MATLAB Function15/1(in), MATLAB Function14/1(in)
  Saturation1/1 -> tau_psi/1, Sum4/1
  Sum9/1 -> Integrator2/1
  MATLAB Function14/1(y) -> Sum8/1
  MATLAB Function11/1(y) -> Sum9/2
  Integrator2/1 -> Sum8/2
  Sum4/1 -> MATLAB Function11/1(e_s_psi)
  K_t_psi/1 -> MATLAB Function11/2(K_t_psi)
  K1/1 -> MATLAB Function15/2(K)
  K2/1 -> MATLAB Function14/2(K)
  MATLAB Function16/1(y) -> Sum8/3
  in/1 -> MATLAB Function16/1(in)
  K/1 -> MATLAB Function16/2(K)

== system simulation_environment_thesis/PHASE 3&4  /PHASE  3/CONTROLLER ==
  v_err : Inport
  psi_err : Inport  Port=2
  speed_0 : Inport  Port=3  PortDimensions=[4,1]
  z_err : Inport  Port=4
  ANTIWINDUP SURGE : Subsystem
  ANTIWINDUP SWAY : Subsystem
  ANTIWINDUP_HEAVE : Subsystem
  ANTIWINDUP_YAW : Subsystem
  Demux : Demux
  MATLAB Function3 : MATLAB Function  TreatAsAtomicUnit=on
  Mux : Mux
  tau : Outport
  wires:
  MATLAB Function3/12(K_i_psi) -> ANTIWINDUP_YAW/1(K1)
  ANTIWINDUP SURGE/1(tau_u) -> Mux/1
  ANTIWINDUP_HEAVE/1(tau_w) -> Mux/3
  ANTIWINDUP_YAW/1(tau_psi) -> Mux/4
  MATLAB Function3/1(K_i_u) -> ANTIWINDUP SURGE/2(K)
  MATLAB Function3/3(K_t_u) -> ANTIWINDUP SURGE/4(K_t_u)
  MATLAB Function3/2(K_p_u) -> ANTIWINDUP SURGE/3(K1)
  speed_0/1 -> MATLAB Function3/1(speed_0)
  Demux/1 -> ANTIWINDUP SURGE/1(in)
  MATLAB Function3/14(K_t_psi) -> ANTIWINDUP_YAW/3(K_t_psi)
  MATLAB Function3/11(K_p_psi) -> ANTIWINDUP_YAW/2(K2)
  ANTIWINDUP SWAY/1(tau_v) -> Mux/2
  v_err/1 -> Demux/1
  Mux/1 -> tau/1
  psi_err/1 -> ANTIWINDUP_YAW/4(in1)
  Demux/2 -> ANTIWINDUP SWAY/1(in)
  MATLAB Function3/4(K_i_v) -> ANTIWINDUP SWAY/2(K)
  MATLAB Function3/5(K_p_v) -> ANTIWINDUP SWAY/3(K1)
  MATLAB Function3/6(K_t_v) -> ANTIWINDUP SWAY/4(K_t_v)
  MATLAB Function3/13(K_d_psi) -> ANTIWINDUP_YAW/6(K)
  Demux/4 -> ANTIWINDUP_YAW/5(in)
  MATLAB Function3/7(K_i_w) -> ANTIWINDUP_HEAVE/1(K1)
  MATLAB Function3/8(K_p_w) -> ANTIWINDUP_HEAVE/2(K2)
  MATLAB Function3/9(K_d_w) -> ANTIWINDUP_HEAVE/6(K)
  MATLAB Function3/10(K_t_w) -> ANTIWINDUP_HEAVE/3(K_t_w)
  Demux/3 -> ANTIWINDUP_HEAVE/5(in)
  z_err/1 -> ANTIWINDUP_HEAVE/4(in1)

== system simulation_environment_thesis/PHASE 3&4  /PHASE  3/REFERENCE GENERATOR ==
  note: nel sistema della DS, non della nave
  eta : Inport
  yaw_est : Inport  Port=2
  v_c : Inport  Port=3
  Constant : Constant  Value=circumference_x  VectorParams1D=off
  Constant1 : Constant  Value=circumference_y  VectorParams1D=off
  Constant2 : Constant  Value=NED_psi_ds + pi
  Constant3 : Constant  Value=50
  Constant4 : Constant  Value=[0;0]  VectorParams1D=off
  Constant5 : Constant  Value=speed_0
  Constant7 : Constant  Value=min_dist_from_ds
  MATLAB Function2 : MATLAB Function  TreatAsAtomicUnit=on
  MATLAB Function3 : MATLAB Function  TreatAsAtomicUnit=on
  MATLAB Function4 : MATLAB Function  TreatAsAtomicUnit=on
  MATLAB Function6 : MATLAB Function  TreatAsAtomicUnit=on
  MATLAB Function7 : MATLAB Function  TreatAsAtomicUnit=on
  Memory1 : Memory
  Memory2 : Memory  InitialCondition=psi_P
  Radians to Degrees : simulink_extras/Transformations/Radians to Degrees
  Scope : Scope
  Scope1 : Scope
  Scope2 : Scope
  desired yaw angle3 : Scope
  yaw_error : Outport
  ref_speed : Outport  Port=2
  wires:
  MATLAB Function3/1(psi) -> MATLAB Function7/2(beta)
  MATLAB Function4/2(direction) -> Memory1/1, MATLAB Function3/1(psi_p), Radians to Degrees/1
  MATLAB Function4/1(y_1) -> MATLAB Function3/2(y_1)
  Memory2/1 -> MATLAB Function4/6("psi_P ")
  Constant7/1 -> MATLAB Function4/5(radius)
  Radians to Degrees/1 -> Scope2/1
  MATLAB Function4/3(flag) -> Scope/1
  Memory1/1 -> MATLAB Function4/7(prev_psi_P)
  MATLAB Function4/4(psi_P) -> Memory2/1
  Constant3/1 -> MATLAB Function3/3(delta_h)
  Constant4/1 -> MATLAB Function4/4(center)
  MATLAB Function2/1(yaw_des) -> MATLAB Function7/4(yaw_dock), MATLAB Function6/1(yaw_ref), desired yaw angle3/1
  Constant5/1 -> MATLAB Function7/3(speed_0)
  Constant/1 -> MATLAB Function4/2(circ_x)
  Constant1/1 -> MATLAB Function4/3(circ_y)
  MATLAB Function7/1(speed) -> ref_speed/1
  yaw_est/1 -> MATLAB Function6/2(yaw_est)
  MATLAB Function4/5(i) -> Scope1/1
  MATLAB Function6/1(yaw_error) -> yaw_error/1
  v_c/1 -> MATLAB Function7/1(v_c)
  eta/1 -> MATLAB Function4/1(eta)
  Constant2/1 -> MATLAB Function2/1("yaw_des ")

== system simulation_environment_thesis/PHASE 3&4  /PHASE  3/dynamic + kinematic models1 ==
  note: body frame
  note: ds frame
  note: body frame
  tau : Inport
  ref speed : Inport  Port=2
  last_yaw__from_ship : Inport  Port=3
  yaw_est : Inport  Port=4
  ds_p_auv : Inport  Port=5
  Constant : Constant  Value=0
  Demux : Demux
  Integrator1 : Integrator  InitialConditionSource=external
  Integrator2 : Integrator  InitialConditionSource=external
  Integrator4 : Integrator  InitialConditionSource=external  InitialCondition=speed_0  UpperSaturationLimit=10^3  LowerSaturationLimit=-10^3
  Integrator5 : Integrator  InitialCondition=const_current
  MATLAB Function : MATLAB Function  TreatAsAtomicUnit=on
  MATLAB Function7 : MATLAB Function  TreatAsAtomicUnit=on
  MATLAB Function9 : MATLAB Function  TreatAsAtomicUnit=on
  Mux : Mux  Inputs=2
  Mux1 : Mux  Inputs=2
  Mux3 : Mux  Inputs=3
  Mux4 : Mux
  Random Number : RandomNumber  Variance=csi1_2^2  Seed=104258147  SampleTime=dt
  Random Number1 : RandomNumber  Variance=csi3_2^2  Seed=1249263617  SampleTime=dt
  Random Number10 : RandomNumber  Variance=csi8_2^2  Seed=990974496  SampleTime=dt
  Random Number3 : RandomNumber  Variance=csi6_2^2  Seed=1982984911  SampleTime=dt
  Random Number4 : RandomNumber  Variance=csi4_2^2  Seed=36455779  SampleTime=dt
  Random Number5 : RandomNumber  Variance=csi2_2^2  Seed=1118814163  SampleTime=dt
  Random Number8 : RandomNumber  Variance=csi5_2^2  Seed=1837104066  SampleTime=dt
  Random Number9 : RandomNumber  Variance=csi7_2^2  Seed=58703202  SampleTime=dt
  Selector1 : Selector  InputPortWidth=4  IndexOptions=Index vector (dialog)  Indices=[4]  OutputSizes=1
  Selector2 : Selector  InputPortWidth=4  IndexOptions=Index vector (dialog)  Indices=[1 2 3]  OutputSizes=1
  Selector3 : Selector  InputPortWidth=4  IndexOptions=Index vector (dialog)  Indices=[1 2 3]  OutputSizes=1
  Sum{,1..2,5} (4 alike) : Sum  Inputs=|++
  Sum4 : Sum  Inputs=|+-
  error speed : Outport
  v_tot ds : Outport  Port=2
  p : Outport  Port=3
  yaw : Outport  Port=4
  body speed : Outport  Port=5
  wires:
  ref speed/1 -> Integrator4/2, Sum4/1
  MATLAB Function9/1(eta_dot) -> Sum5/1, Demux/1
  tau/1 -> MATLAB Function7/2(tau)
  Sum4/1 -> error speed/1
  Sum5/1 -> Selector3/1, v_tot ds/1
  Integrator4/1 -> MATLAB Function9/2(speed), MATLAB Function7/1(speed), Sum4/2, body speed/1
  Integrator5/1 -> Sum5/2
  last_yaw__from_ship/1 -> Integrator1/2
  Integrator1/1 -> MATLAB Function/1("angle ")
  yaw_est/1 -> MATLAB Function9/1(yaw)
  MATLAB Function/1(angle) -> Mux/2, yaw/1
  ds_p_auv/1 -> Integrator2/2
  Random Number1/1 -> Mux3/3
  Mux3/1 -> Sum/2
  Sum/1 -> Integrator2/1
  Random Number5/1 -> Mux3/2
  Random Number/1 -> Mux3/1
  Integrator2/1 -> Mux/1
  Random Number10/1 -> Sum2/2
  Selector1/1 -> Sum2/1
  Selector2/1 -> Mux1/1
  Sum2/1 -> Mux1/2
  Mux1/1 -> Integrator4/1
  MATLAB Function7/1(speed_dot) -> Selector2/1, Selector1/1
  Random Number9/1 -> Sum1/2
  Sum1/1 -> Integrator1/1
  Demux/4 -> Sum1/1
  Random Number8/1 -> Mux4/2
  Random Number4/1 -> Mux4/1
  Mux4/1 -> Integrator5/1
  Random Number3/1 -> Mux4/3
  Selector3/1 -> Sum/1
  Mux/1 -> p/1
  Constant/1 -> Mux4/4

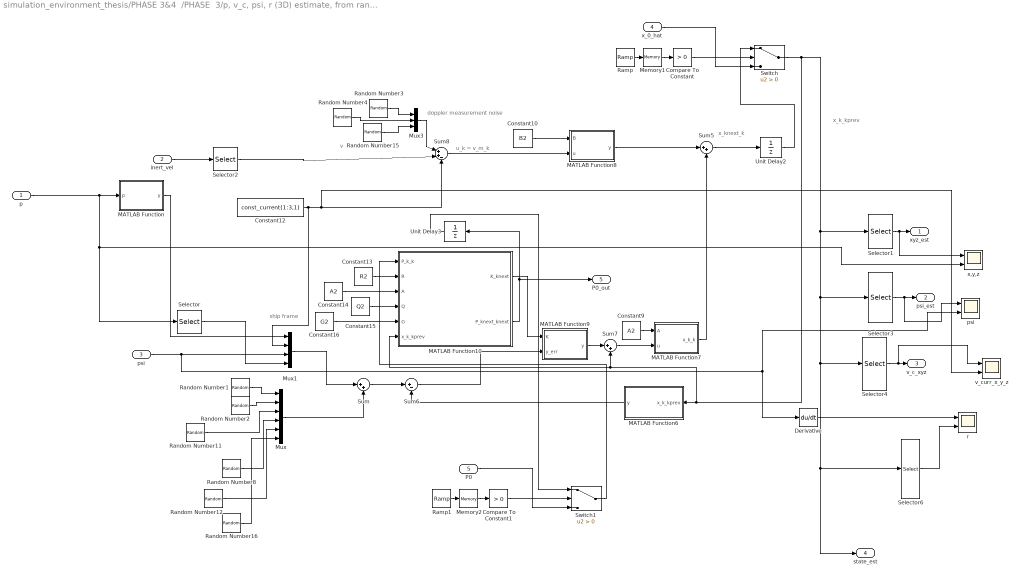
== system simulation_environment_thesis/PHASE 3&4  /PHASE  3/p, v_c, psi, r (3D) estimate, from range, v_c, psi, z measurement ==
  note: x_k_kprev
  note: x_knext_k
  note: ship frame
  note: v
  note: doppler measurement noise
  note: u_k = v_m_k
  p : Inport
  inert_vel : Inport  Port=2
  psi : Inport  Port=3
  x_0_hat : Inport  Port=4
  P0 : Inport  Port=5
  Compare To Constant : simulink/Logic and Bit Operations/Compare To Constant  relop=>  const=0  OutDataTypeStr=boolean
  Compare To Constant1 : simulink/Logic and Bit Operations/Compare To Constant  relop=>  const=0  OutDataTypeStr=boolean
  Constant10 : Constant  Value=B2
  Constant12 : Constant  Value=const_current(1:3,1)  VectorParams1D=off
  Constant13 : Constant  Value=R2
  Constant14 : Constant  Value=A2
  Constant15 : Constant  Value=Q2
  Constant16 : Constant  Value=G2
  Constant9 : Constant  Value=A2  VectorParams1D=off
  Derivative : Derivative
  MATLAB Function : MATLAB Function  TreatAsAtomicUnit=on
  MATLAB Function10 : MATLAB Function  TreatAsAtomicUnit=on
  MATLAB Function6 : MATLAB Function  TreatAsAtomicUnit=on
  MATLAB Function7 : MATLAB Function  TreatAsAtomicUnit=on
  MATLAB Function8 : MATLAB Function  TreatAsAtomicUnit=on
  MATLAB Function9 : MATLAB Function  TreatAsAtomicUnit=on
  Memory1 : Memory
  Memory2 : Memory
  Mux : Mux  Inputs=6
  Mux1 : Mux
  Mux3 : Mux  Inputs=3
  Ramp : simulink/Sources/Ramp  slope=1  start=0  InitialOutput=0
  Ramp1 : simulink/Sources/Ramp  slope=1  start=0  InitialOutput=0
  Random Number1 : RandomNumber  Variance=eta1_2^2  Seed=779859847  SampleTime=dt
  Random Number11 : RandomNumber  Variance=eta3_2^2  Seed=1688645331  SampleTime=dt
  Random Number12 : RandomNumber  Variance=eta5_2^2  Seed=2100594210  SampleTime=dt
  Random Number15 : RandomNumber  Variance=var_vm_z^2  Seed=1407452486  SampleTime=dt
  Random Number16 : RandomNumber  Variance=eta6_2^2  Seed=1934344127  SampleTime=dt
  Random Number2 : RandomNumber  Variance=eta2_2^2  Seed=1890086545  SampleTime=dt
  Random Number3 : RandomNumber  Variance=var_vm_x^2  Seed=536965506  SampleTime=dt
  Random Number4 : RandomNumber  Variance=var_vm_y^2  Seed=2026222191  SampleTime=dt
  Random Number8 : RandomNumber  Variance=eta4_2^2  Seed=1886161357  SampleTime=dt
  Selector : Selector  InputPortWidth=3  IndexOptions=Index vector (dialog)  Indices=[3]  OutputSizes=1
  Selector1 : Selector  InputPortWidth=8  IndexOptions=Index vector (dialog)  Indices=[1 2 3]  OutputSizes=1
  Selector2 : Selector  InputPortWidth=4  IndexOptions=Index vector (dialog)  Indices=[1 2 3]  OutputSizes=1
  Selector3 : Selector  InputPortWidth=8  IndexOptions=Index vector (dialog)  Indices=[7]  OutputSizes=1
  Selector4 : Selector  InputPortWidth=8  IndexOptions=Index vector (dialog)  Indices=[4 5 6]  OutputSizes=1
  Selector6 : Selector  InputPortWidth=8  IndexOptions=Index vector (dialog)  Indices=[8]  OutputSizes=1
  Sum : Sum  Inputs=|++
  Sum5 : Sum  Inputs=|++
  Sum6 : Sum  Inputs=|+-
  Sum7 : Sum  Inputs=|++
  Sum8 : Sum  Inputs=|++-
  Switch : Switch  Criteria=u2 > Threshold  SaturateOnIntegerOverflow=off  InputSameDT=off
  Switch1 : Switch  Criteria=u2 > Threshold  SaturateOnIntegerOverflow=off  InputSameDT=off
  Unit Delay2 : UnitDelay  SampleTime=-1  HasFrameUpgradeWarning=on
  Unit Delay3 : UnitDelay  InitialCondition=alpha2*eye(8)  SampleTime=-1  HasFrameUpgradeWarning=on
  "psi " : Scope  NumInputPorts=2
  r : Scope  NumInputPorts=2
  v_curr_x_y_z : Scope  NumInputPorts=2
  x,y,z : Scope  NumInputPorts=2
  xyz_est : Outport
  psi_est : Outport  Port=2
  v_c_xyz : Outport  Port=3
  state_est : Outport  Port=4
  P0_out : Outport  Port=5
  wires:
  MATLAB Function7/1(x_k_k) -> Sum5/2
  Constant14/1 -> MATLAB Function10/3(A)
  Sum7/1 -> MATLAB Function7/2(u)
  Random Number4/1 -> Mux3/2
  MATLAB Function8/1(y) -> Sum5/1
  Random Number3/1 -> Mux3/1
  Constant15/1 -> MATLAB Function10/4(Q)
  MATLAB Function9/1(y) -> Sum7/1
  Sum5/1 -> Unit Delay2/1
  MATLAB Function10/2(P_knext_knext) -> P0_out/1, Unit Delay3/1
  Sum8/1 -> MATLAB Function8/2(u)
  MATLAB Function10/1(K_knext) -> MATLAB Function9/1(K)
  p/1 -> Selector/1, x,y,z/2, MATLAB Function/1(p)
  Constant9/1 -> MATLAB Function7/1(A)
  Constant13/1 -> MATLAB Function10/2(R)
  Sum6/1 -> MATLAB Function9/2(y_err)
  Constant10/1 -> MATLAB Function8/1(B)
  Mux3/1 -> Sum8/1
  Constant16/1 -> MATLAB Function10/5(G)
  Sum/1 -> Sum6/1
  MATLAB Function6/1(y) -> Sum6/2
  Selector1/1 -> x,y,z/1, xyz_est/1
  psi/1 -> "psi "/2, Derivative/1, Mux1/3
  Mux1/1 -> Sum/1
  Selector3/1 -> "psi "/1, psi_est/1
  Selector4/1 -> v_curr_x_y_z/1, v_c_xyz/1
  inert_vel/1 -> Selector2/1
  Mux/1 -> Sum/2
  Random Number2/1 -> Mux/2
  Random Number11/1 -> Mux/3
  Random Number1/1 -> Mux/1
  MATLAB Function/1(y) -> Mux1/1
  Constant12/1 -> Mux1/2, Sum8/3, v_curr_x_y_z/2
  Random Number8/1 -> Mux/4
  Random Number12/1 -> Mux/5
  Derivative/1 -> r/1
  Selector6/1 -> r/2
  Random Number15/1 -> Mux3/3
  Selector2/1 -> Sum8/2
  Random Number16/1 -> Mux/6
  Selector/1 -> Mux1/4
  Switch/1 -> Selector3/1, Selector4/1, state_est/1, Selector6/1, Selector1/1, MATLAB Function10/6(x_k_kprev), Sum7/2, MATLAB Function6/1(x_k_kprev)
  Ramp/1 -> Memory1/1
  Memory1/1 -> Compare To Constant/1
  Compare To Constant/1 -> Switch/2
  x_0_hat/1 -> Switch/3
  Unit Delay2/1 -> Switch/1
  Unit Delay3/1 -> Switch1/1
  Switch1/1 -> MATLAB Function10/1(P_k_k)
  Memory2/1 -> Compare To Constant1/1
  Compare To Constant1/1 -> Switch1/2
  Ramp1/1 -> Memory2/1
  P0/1 -> Switch1/3
